simulink model "wlan_phy_rx_pmd"  (Simulink 7.8)
settings: Solver=VariableStepDiscrete  SolverMode=SingleTasking  StartTime=0.0  StopTime=rx_sim_time  RelTol=1e-3
PostLoadFcn:
  | wlan_phy_rx_init
InitFcn:
  | wlan_phy_rx_init
StartFcn:
  | wlan_phy_rx_init
values from neighbouring files (symbols this model uses):
  barker_seq20 = 0.95 * circshift(barker_seq20, [0 4]) ./ max(abs(barker_seq20))    [wlan_phy_rx_init.m]
  bit_scrambler_lfsr_bytes = bi2de(reshape(repmat(scr, 1, 8), 8, 127)', 'left-msb')    [wlan_phy_rx_init.m]
  deinterleave_ROM = [deinterleave_ROM interleave_64QAM zeros(1, 512-length(interleave_64QAM))]    [wlan_phy_rx_init.m]
  longCorr_coef_i = [3*real(longCorr_coef)]    [wlan_phy_rx_init.m]
  longCorr_coef_q = [3*imag(longCorr_coef)]    [wlan_phy_rx_init.m]
  MAX_CP_LEN = 32    [wlan_phy_rx_init.m]
  MAX_NCBPS = NCBPS_64QAM    (= 288)    [wlan_phy_rx_init.m]
  MAX_NUM_BYTES = 4096    [wlan_phy_rx_init.m]
  MAX_NUM_SC = 64    [wlan_phy_rx_init.m]
  MAX_NUM_SYMS = 600    [wlan_phy_rx_init.m]
  NCBPS_16QAM = 192    [wlan_phy_rx_init.m]
  NCBPS_64QAM = 288    [wlan_phy_rx_init.m]
  NCBPS_BPSK = 48    [wlan_phy_rx_init.m]
  NCBPS_QPSK = 96    [wlan_phy_rx_init.m]
  NDBPS_16QAM12 = 96    [wlan_phy_rx_init.m]
  NDBPS_16QAM34 = 144    [wlan_phy_rx_init.m]
  NDBPS_64QAM23 = 192    [wlan_phy_rx_init.m]
  NDBPS_64QAM34 = 216    [wlan_phy_rx_init.m]
  NDBPS_BPSK12 = 24    [wlan_phy_rx_init.m]
  NDBPS_BPSK34 = 36    [wlan_phy_rx_init.m]
  NDBPS_QPSK12 = 48    [wlan_phy_rx_init.m]
  NDBPS_QPSK34 = 72    [wlan_phy_rx_init.m]
  PHY_MIN_PKT_LEN = 14    [wlan_phy_rx_init.m]
  preFFT_sampBuff_numSamps = 4*MAX_NUM_SC    (= 256)    [wlan_phy_rx_init.m]
  rx_sim_sig_samp_time = 8    [wlan_phy_rx_init.m]
  scr_ind_rev = zeros(1,128)    [wlan_phy_rx_init.m]
undefined symbols (not defined in the file or in its folder): gcbh, xlBlockLoadCallback, xlGetNormalizedPeriod, xlipgetpartsetting
libraries: xbsIndex_r4 x3524, simulink x1, xbsEDKLib_r4 x1
(part 10 of 21)

== system wlan_phy_rx_pmd/Detect & Decode/Soft Demap/16QAM Soft De-Map ==
  note: 16-QAM Demap:
      | Each sym is [b0 b1 b2 b3]
      | b0 = 1 if (I > 0)
      | b1 = 1 if(abs(I) < 2/sqrt(10))
      | b2 = 1 if(Q > 0)
      | b3 = 1 if(abs(Q) < 2/sqrt(10))
  IQ Valid : Inport
  I : Inport  Port=2
  Q : Inport  Port=3
  Reest : Inport  Port=4
  Absolute : xbsIndex_r4/Absolute  precision=Full  arith_type=Unsigned  n_bits=8  bin_pt=0  quantization=Truncate  overflow=Saturate  en=off  latency=0  dbl_ovrd=off  block_type=abs
  AddSub : xbsIndex_r4/AddSub  mode=Subtraction  use_carryin=off  use_carryout=off  en=off  latency=0  precision=User Defined  arith_type="Signed  (2's comp)"  n_bits=8  bin_pt=7  quantization=Truncate  overflow=Saturate  dbl_ovrd=off  use_behavioral_HDL=off  hw_selection=Fabric  pipelined=off  block_type=addsub
  Constant : xbsIndex_r4/Constant  const=2/sqrt(10)  gui_display_data_type=Fixed-point  arith_type=Unsigned  n_bits=12  bin_pt=12  preci_type=Single  exp_width=8  frac_width=24  explicit_period=off  period=1  equ=P=C  opselect=C  inp2=PCIN>>17  opr=+  inp1=P  carry=CIN  dbl_ovrd=off  block_type=constant
  Delay2 : xbsIndex_r4/Delay  rst=off  infoeditControl=Selection of Reset will increase slice count due to use of real FFs and instead of SRLs  en=off  latency=1  dbl_ovrd=off  reg_retiming=off  block_type=delay
  Inverter : xbsIndex_r4/Inverter  en=off  latency=0  dbl_ovrd=off  block_type=inv
      settings: xl_area=[0 0 0 0 0 0 0]  block_version=VER_STRING_GOES_HERE
  Logical1 : xbsIndex_r4/Logical  logical_function=OR  inputs=2  en=off  latency=0  precision=Full  arith_type=Unsigned  n_bits=16  bin_pt=0  align_bp=on  dbl_ovrd=off  block_type=logical
  Logical2 : xbsIndex_r4/Logical  logical_function=OR  inputs=2  en=off  latency=0  precision=Full  arith_type=Unsigned  n_bits=16  bin_pt=0  align_bp=on  dbl_ovrd=off  block_type=logical
  Mux : xbsIndex_r4/Mux  inputs=2  en=off  latency=0  precision=Full  arith_type=Unsigned  n_bits=16  bin_pt=14  quantization=Truncate  overflow=Wrap  dbl_ovrd=off  block_type=mux
  Register1 : xbsIndex_r4/Register  init=0  rst=off  en=on  dbl_ovrd=off  block_type=register
  S-R Latch1 : Subsystem
  Ready : Outport
  Bit A : Outport  Port=2
  Bit B : Outport  Port=3
  Bits Valid : Outport  Port=4
  wires:
  IQ Valid/1 -> S-R Latch1/1(S), Register1/2, Logical2/1, Delay2/1
  S-R Latch1/1(Q) -> Inverter/1, Mux/1, Logical1/2
  Inverter/1 -> Ready/1
  Q/1 -> Register1/1
  Constant/1 -> AddSub/1
  Absolute/1 -> AddSub/2
  Mux/1 -> Bit A/1, Absolute/1
  I/1 -> Mux/2
  Register1/1 -> Mux/3
  AddSub/1 -> Bit B/1
  Delay2/1 -> Logical2/2
  Logical2/1 -> Bits Valid/1
  Logical1/1 -> S-R Latch1/2(R)
  Reest/1 -> Logical1/1

== system wlan_phy_rx_pmd/Detect & Decode/Soft Demap/64QAM Soft De-Map ==
  note: 64-QAM Demap:
      | Each sym is [b0 b1 b2 b3 b4 b5]
      | b0 = 1 if (I > 0)
      | b1 = 1 if(abs(I) < 4/sqrt(42))
      |
      | b2 = 1 if(2/sqrt(42) < abs(I) < 6/sqrt(42))
      | b3 = 1 if(Q > 0)
      |
      | b4 = 1 if(abs(Q) < 4/sqrt(42))
      | b5 = 1 if(2/sqrt(42) < abs(Q) < 6/sqrt(42))
  IQ Valid : Inport
  I : Inport  Port=2
  Q : Inport  Port=3
  Reest : Inport  Port=4
  64QAM Demap : Scope  NumInputPorts=8
      settings: TimeRange=20000  YMin=0~-1~0~0~-1~0~-1~-1  YMax=1~1~1~1~1~1~1~1  SaveName=ScopeData26
  Ctrl : Subsystem
  Gateway Out{1..6} (6 alike) : xbsIndex_r4/Gateway Out  inherit_from_input=off  hdl_port=off  timing_constraint=None  locs_specified=off  LOCs={}  UseAsDAC=off  DACChannel='1'  block_type=gatewayout
  Logical3 : xbsIndex_r4/Logical  logical_function=AND  inputs=2  en=off  latency=0  precision=Full  arith_type=Unsigned  n_bits=16  bin_pt=0  align_bp=on  dbl_ovrd=off  block_type=logical
  Mux : xbsIndex_r4/Mux  inputs=3  en=off  latency=0  precision=Full  arith_type=Unsigned  n_bits=16  bin_pt=14  quantization=Truncate  overflow=Wrap  dbl_ovrd=off  block_type=mux
  Mux1 : xbsIndex_r4/Mux  inputs=3  en=off  latency=0  precision=Full  arith_type=Unsigned  n_bits=16  bin_pt=14  quantization=Truncate  overflow=Wrap  dbl_ovrd=off  block_type=mux
  Register2 : xbsIndex_r4/Register  init=0  rst=off  en=on  dbl_ovrd=off  block_type=register
  Register3 : xbsIndex_r4/Register  init=0  rst=off  en=on  dbl_ovrd=off  block_type=register
  b1/b4 map : Subsystem
  b1/b4 map1 : Subsystem
  b2/b5 map : Subsystem
  b2/b5 map1 : Subsystem
  Ready : Outport
  Bit A : Outport  Port=2
  Bit B : Outport  Port=3
  Bits Valid : Outport  Port=4
  wires:
  I/1 -> Register2/1, Gateway Out3/1
  Mux/1 -> Bit A/1
  b1/b4 map/1(b) -> Mux1/2
  Logical3/1 -> Register2/2, Register3/2
  IQ Valid/1 -> Logical3/2, Ctrl/1(Vin), Gateway Out1/1
  b2/b5 map/1(b) -> Mux/3
  Register2/1 -> b2/b5 map/1(IQ), Mux/2, b1/b4 map/1(IQ)
  Register3/1 -> Mux1/3, b1/b4 map1/1(IQ), b2/b5 map1/1(IQ)
  Q/1 -> Register3/1, Gateway Out2/1
  b1/b4 map1/1(b) -> Mux/4
  b2/b5 map1/1(b) -> Mux1/4
  Mux1/1 -> Bit B/1
  Ctrl/3(Sel) -> Mux/1, Mux1/1, Gateway Out4/1
  Ctrl/2(Vout) -> Bits Valid/1, Gateway Out5/1
  Ctrl/1(Ready) -> Logical3/1, Ready/1, Gateway Out6/1
  Gateway Out1/1 -> 64QAM Demap/1  "valid in"
  Gateway Out3/1 -> 64QAM Demap/2  "I In"
  Gateway Out2/1 -> 64QAM Demap/3  "Q In"
  Gateway Out4/1 -> 64QAM Demap/4  "Mux Sel"
  Gateway Out5/1 -> 64QAM Demap/5  "Vout"
  Gateway Out6/1 -> 64QAM Demap/6  "Ready"
  Reest/1 -> Ctrl/2(Reest)

== system wlan_phy_rx_pmd/Detect & Decode/Soft Demap/BPSK Soft De-Map ==
  V_in : Inport
  I : Inport  Port=2
  Q : Inport  Port=3
  Reest : Inport  Port=4
  Constant : xbsIndex_r4/Constant  const=1  gui_display_data_type=Boolean  arith_type=Boolean  n_bits=16  bin_pt=14  preci_type=Single  exp_width=8  frac_width=24  explicit_period=on  period=1  equ=P=C  opselect=C  inp2=PCIN>>17  opr=+  inp1=P  carry=CIN  dbl_ovrd=off  block_type=constant
  Logical1 : xbsIndex_r4/Logical  logical_function=OR  inputs=2  en=off  latency=0  precision=Full  arith_type=Unsigned  n_bits=16  bin_pt=0  align_bp=on  dbl_ovrd=off  block_type=logical
  Logical2 : xbsIndex_r4/Logical  logical_function=AND  inputs=2  en=off  latency=0  precision=Full  arith_type=Unsigned  n_bits=16  bin_pt=0  align_bp=on  dbl_ovrd=off  block_type=logical
  Register2 : xbsIndex_r4/Register  init=0  rst=off  en=on  dbl_ovrd=off  block_type=register
  S-R Latch1 : Subsystem
  Ready : Outport
  Bit A : Outport  Port=2
  Bit B : Outport  Port=3
  Bits Valid : Outport  Port=4
  wires:
  I/1 -> Register2/1, Bit B/1
  Constant/1 -> Ready/1
  V_in/1 -> S-R Latch1/1(S), Register2/2, Logical2/2
  S-R Latch1/1(Q) -> Logical2/1
  Register2/1 -> Bit A/1
  Logical2/1 -> Bits Valid/1, Logical1/2
  Logical1/1 -> S-R Latch1/2(R)
  Reest/1 -> Logical1/1

== system wlan_phy_rx_pmd/Detect & Decode/Soft Demap/QPSK Soft De-Map ==
  IQ Valid : Inport
  I : Inport  Port=2
  Q : Inport  Port=3
  Reest : Inport  Port=4
  Constant1 : xbsIndex_r4/Constant  const=1  gui_display_data_type=Fixed-point  arith_type=Boolean  n_bits=1  bin_pt=0  preci_type=Single  exp_width=8  frac_width=24  explicit_period=off  period=1  equ=P=C  opselect=C  inp2=PCIN>>17  opr=+  inp1=P  carry=CIN  dbl_ovrd=off  block_type=constant
      settings: block_version=VER_STRING_GOES_HERE
  Ready : Outport
  Bit A : Outport  Port=2
  Bit B : Outport  Port=3
  Bits Valid : Outport  Port=4
  wires:
  I/1 -> Bit A/1
  Q/1 -> Bit B/1
  IQ Valid/1 -> Bits Valid/1
  Constant1/1 -> Ready/1
  Reest/1 -> ?

== system wlan_phy_rx_pmd/Detect & Decode/Soft Demap/Scale & Quantize ==
  Bit A : Inport
  Bit B : Inport  Port=2
  Bits Valid : Inport  Port=3
  mod_sel : Inport  Port=4
  Delay : xbsIndex_r4/Delay  rst=off  infoeditControl=Selection of Reset will increase slice count due to use of real FFs and instead of SRLs  en=off  latency=1  dbl_ovrd=off  reg_retiming=off  block_type=delay
  Delay1 : xbsIndex_r4/Delay  rst=off  infoeditControl=Selection of Reset will increase slice count due to use of real FFs and instead of SRLs  en=off  latency=1  dbl_ovrd=off  reg_retiming=off  block_type=delay
  Display : Display
  Display1 : Display
  Display2 : Display
  Gateway Out{1..8} (8 alike) : xbsIndex_r4/Gateway Out  inherit_from_input=off  hdl_port=off  timing_constraint=None  locs_specified=off  LOCs={}  UseAsDAC=off  DACChannel='1'  block_type=gatewayout
  Mult1 : xbsIndex_r4/Mult  precision=User Defined  arith_type="Signed  (2's comp)"  n_bits=13  bin_pt=0  quantization=Truncate  overflow=Saturate  en=off  latency=1  dbl_ovrd=off  use_behavioral_HDL=off  opt=Speed  use_embedded=on  optimum_pipeline=off  pipeline=on  use_rpm=on  placement_style=Triangular  block_type=mult
  Mult2 : xbsIndex_r4/Mult  precision=User Defined  arith_type="Signed  (2's comp)"  n_bits=13  bin_pt=0  quantization=Truncate  overflow=Saturate  en=off  latency=1  dbl_ovrd=off  use_behavioral_HDL=off  opt=Speed  use_embedded=on  optimum_pipeline=off  pipeline=on  use_rpm=on  placement_style=Triangular  block_type=mult
  Mux : xbsIndex_r4/Mux  inputs=4  en=off  latency=0  precision=Full  arith_type=Unsigned  n_bits=16  bin_pt=14  quantization=Truncate  overflow=Wrap  dbl_ovrd=off  block_type=mux
  Negate : xbsIndex_r4/Negate  precision=User Defined  arith_type="Signed  (2's comp)"  n_bits=4  bin_pt=0  quantization=Truncate  overflow=Saturate  en=off  latency=0  dbl_ovrd=off  block_type=negate
  Negate1 : xbsIndex_r4/Negate  precision=User Defined  arith_type="Signed  (2's comp)"  n_bits=4  bin_pt=0  quantization=Truncate  overflow=Saturate  en=off  latency=0  dbl_ovrd=off  block_type=negate
  Quantize : Subsystem
  Quantize1 : Subsystem
  Reinterpret : xbsIndex_r4/Reinterpret  force_arith_type=off  arith_type="Signed  (2's comp)"  force_bin_pt=on  bin_pt=0  block_type=reinterpret
  Reinterpret1 : xbsIndex_r4/Reinterpret  force_arith_type=off  arith_type="Signed  (2's comp)"  force_bin_pt=on  bin_pt=0  block_type=reinterpret
  Scale/Quant : Scope  NumInputPorts=8
      settings: TimeRange=178.0593691607082  YMin=-1~-95.55~5.7~-573.3~-4.2~-1~-1~-1  YMax=1~-86.45~6.3~-518.7~-3.8~1~1~1  SaveName=ScopeData2
  LLR A : Outport
  LLR B : Outport  Port=2
  LLRs Valid : Outport  Port=3
  " mod_sel" : Outport  Port=4
  wires:
  mod_sel/1 -> Mux/1, Delay1/1
  [regRx_FEC_SCALE_QPSK] -> Mux/3, Mux/2, Gateway Out6/1
  [regRx_FEC_SCALE_16QAM] -> Mux/4, Gateway Out7/1
  [regRx_FEC_SCALE_64QAM] -> Mux/5, Gateway Out8/1
  Bit A/1 -> Reinterpret/1
  Mult1/1 -> Quantize/1(D), Gateway Out1/1
  Quantize/1(Q) -> Gateway Out5/1, Negate/1
  Mux/1 -> Mult1/2, Gateway Out4/1, Mult2/2
  Bits Valid/1 -> Delay/1, Gateway Out2/1
  Delay/1 -> LLRs Valid/1
  Delay1/1 -> " mod_sel"/1
  Gateway Out2/1 -> Scale/Quant/1  "In Valid"
  Gateway Out3/1 -> Scale/Quant/2  "Soft Bit In"
  Gateway Out4/1 -> Scale/Quant/3  "Scale"
  Gateway Out1/1 -> Scale/Quant/4  "Bit Scaled"
  Gateway Out5/1 -> Scale/Quant/5  "LLR"
  Reinterpret/1 -> Mult1/1, Gateway Out3/1
  Negate/1 -> LLR A/1
  Gateway Out8/1 -> Display2/1
  Gateway Out7/1 -> Display1/1
  Gateway Out6/1 -> Display/1
  Bit B/1 -> Reinterpret1/1
  Reinterpret1/1 -> Mult2/1
  Mult2/1 -> Quantize1/1(D)
  Quantize1/1(Q) -> Negate1/1
  Negate1/1 -> LLR B/1

== system wlan_phy_rx_pmd/Detect & Decode/Sym FIFO/S-R Latch1 ==
  S : Inport
  R : Inport  Port=2
  Constant1 : xbsIndex_r4/Constant  const=1  gui_display_data_type=Fixed-point  arith_type=Boolean  n_bits=1  bin_pt=0  preci_type=Single  exp_width=8  frac_width=24  explicit_period=off  period=1  equ=P=C  opselect=C  inp2=PCIN>>17  opr=+  inp1=P  carry=CIN  dbl_ovrd=off  block_type=constant
      settings: block_version=VER_STRING_GOES_HERE
  Convert1 : xbsIndex_r4/Convert  gui_display_data_type=Boolean  arith_type=Boolean  n_bits=1  bin_pt=0  float_type=Single  exp_bits=8  fraction_bits=24  quantization=Truncate  overflow=Wrap  en=off  latency=0  dbl_ovrd=off  pipeline=off  block_type=convert
  Convert2 : xbsIndex_r4/Convert  gui_display_data_type=Boolean  arith_type=Boolean  n_bits=1  bin_pt=0  float_type=Single  exp_bits=8  fraction_bits=24  quantization=Truncate  overflow=Wrap  en=off  latency=0  dbl_ovrd=off  pipeline=off  block_type=convert
  Register2 : xbsIndex_r4/Register  init=0  rst=on  en=on  dbl_ovrd=off  block_type=register
      settings: xl_area=[1 1 0 0 0 0 0]  block_version=VER_STRING_GOES_HERE
  Q : Outport
  wires:
  S/1 -> Convert2/1
  Register2/1 -> Q/1
  R/1 -> Convert1/1
  Constant1/1 -> Register2/1
  Convert1/1 -> Register2/2
  Convert2/1 -> Register2/3

== system wlan_phy_rx_pmd/FCS & Pkt Buf/FCS Calc/CRC32 Calc ==
  byte_valid : Inport
  byte : Inport  Port=2
  rst : Inport  Port=3
  24LSB : xbsIndex_r4/Slice  nbits=24  boolean_output=off  mode=Lower Bit Location + Width  bit1=0  base1=MSB of Input  bit0=0  base0=LSB of Input  dbl_ovrd=off  block_type=slice
      settings: infoedit="Extracts a given range of bits from each input sample and presents it at the output.  The output type is ordinarily unsigned with binary point at zero, but can be Boolean when the slice is one bit wide.<P><P>Hardware notes: In hardware this block costs nothing."  block_version=VER_STRING_GOES_HERE
  8MSB : xbsIndex_r4/Slice  nbits=8  boolean_output=off  mode=Upper Bit Location + Width  bit1=0  base1=MSB of Input  bit0=0  base0=LSB of Input  dbl_ovrd=off  block_type=slice
      settings: infoedit="Extracts a given range of bits from each input sample and presents it at the output.  The output type is ordinarily unsigned with binary point at zero, but can be Boolean when the slice is one bit wide.<P><P>Hardware notes: In hardware this block costs nothing."  block_version=VER_STRING_GOES_HERE
  Assert1 : xbsIndex_r4/Assert  assert_type=off  type_source=Explicitly  gui_display_data_type=Fixed-point  arith_type="Signed  (2's comp)"  n_bits=16  bin_pt=14  preci_type=Single  exp_width=8  frac_width=24  assert_rate=on  rate_source=Explicitly  period=1  output_port=on  block_type=assert
      settings: block_version=VER_STRING_GOES_HERE
  CRC Remainders1 : xbsIndex_r4/ROM  depth=256  initVector=CRC_Table32  distributed_mem=Distributed memory  rst=off  init_reg=0  en=off  latency=0  gui_display_data_type=Fixed-point  arith_type=Unsigned  n_bits=32  bin_pt=0  preci_type=Single  exp_width=8  frac_width=24  dbl_ovrd=off  optimize=Area  use_rpm=off  block_type=sprom
      settings: xl_area=[0, 0, 0, 0, 0, 0, 0]  block_version=VER_STRING_GOES_HERE
  Concat1 : xbsIndex_r4/Concat  num_inputs=2  dbl_ovrd=off  block_type=concat
      settings: block_version=VER_STRING_GOES_HERE
  Constant1 : xbsIndex_r4/Constant  const=0  gui_display_data_type=Fixed-point  arith_type=Unsigned  n_bits=8  bin_pt=0  preci_type=Single  exp_width=8  frac_width=24  explicit_period=off  period=1  equ=P=C  opselect=C  inp2=PCIN>>17  opr=+  inp1=P  carry=CIN  dbl_ovrd=off  block_type=constant
      settings: block_version=VER_STRING_GOES_HERE
  Constant3 : xbsIndex_r4/Constant  const=hex2dec('C704DD7B')  gui_display_data_type=Fixed-point  arith_type=Unsigned  n_bits=32  bin_pt=0  preci_type=Single  exp_width=8  frac_width=24  explicit_period=off  period=1  equ=P=C  opselect=C  inp2=PCIN>>17  opr=+  inp1=P  carry=CIN  dbl_ovrd=off  block_type=constant
  Endian Swap : Subsystem
  FCS Calc : Scope  NumInputPorts=8
      settings: TimeRange=10000  YMin=0~0~10~-1~-1~-1~-1~-1  YMax=1~1~120~1~1~1~1~1  SaveName=ScopeData15
  Gateway Out{1..3,10} (4 alike) : xbsIndex_r4/Gateway Out  inherit_from_input=off  hdl_port=off  timing_constraint=None  locs_specified=off  LOCs={}  UseAsDAC=off  DACChannel='1'  block_type=gatewayout
  Logical3 : xbsIndex_r4/Logical  logical_function=XOR  inputs=2  en=off  latency=0  precision=Full  arith_type=Unsigned  n_bits=8  bin_pt=2  align_bp=on  dbl_ovrd=off  block_type=logical
      settings: xl_area=[0, 0, 0, 0, 0, 0, 0]  block_version=VER_STRING_GOES_HERE
  Logical4 : xbsIndex_r4/Logical  logical_function=XOR  inputs=2  en=off  latency=0  precision=Full  arith_type=Unsigned  n_bits=8  bin_pt=2  align_bp=on  dbl_ovrd=off  block_type=logical
      settings: xl_area=[0, 0, 0, 0, 0, 0, 0]  block_version=VER_STRING_GOES_HERE
  Relational1 : xbsIndex_r4/Relational  mode=a=b  en=off  latency=0  dbl_ovrd=off  block_type=relational
  crc_accum : xbsIndex_r4/Register  init=hex2dec('FFFFFFFF')  rst=on  en=on  dbl_ovrd=off  block_type=register
      settings: xl_area=[0, 0, 0, 0, 0, 0, 0]  block_version=VER_STRING_GOES_HERE
  FCS Good : Outport
  wires:
  Endian Swap/1(I) -> Logical3/2
  Logical3/1 -> CRC Remainders1/1
  CRC Remainders1/1 -> Logical4/2
  Concat1/1 -> Logical4/1
  Constant1/1 -> Concat1/2
  Logical4/1 -> crc_accum/1
  crc_accum/1 -> Assert1/1
  Assert1/1 -> 24LSB/1, 8MSB/1, Relational1/1
  byte_valid/1 -> crc_accum/3, Gateway Out10/1
  rst/1 -> crc_accum/2, Gateway Out1/1
  24LSB/1 -> Concat1/1
  8MSB/1 -> Logical3/1
  byte/1 -> Endian Swap/1(B), Gateway Out2/1
  Constant3/1 -> Relational1/2
  Relational1/1 -> FCS Good/1, Gateway Out3/1
  Gateway Out10/1 -> FCS Calc/1  "Vin"
  Gateway Out1/1 -> FCS Calc/2  "Reset"
  Gateway Out2/1 -> FCS Calc/3  "Byte"
  Gateway Out3/1 -> FCS Calc/4  "Good"

== system wlan_phy_rx_pmd/FCS & Pkt Buf/FCS Calc/Posedge ==
  D : Inport
  Delay : xbsIndex_r4/Delay  rst=off  infoeditControl=Selection of Reset will increase slice count due to use of real FFs and instead of SRLs  en=off  latency=1  dbl_ovrd=off  reg_retiming=off  block_type=delay
      settings: infoedit=Hardware notes: A delay line is a chain, each link of which is an SRL16 followed by a flip-flop. If register retiming is enabled, the delay line is a chain of flip-flops.  xl_area=[1 1 0 0 0 0 0]  block_version=VER_STRING_GOES_HERE
  Inverter : xbsIndex_r4/Inverter  en=off  latency=0  dbl_ovrd=off  block_type=inv
      settings: xl_area=[0 0 0 0 0 0 0]  block_version=VER_STRING_GOES_HERE
  Logical1 : xbsIndex_r4/Logical  logical_function=AND  inputs=2  en=off  latency=0  precision=Full  arith_type=Unsigned  n_bits=8  bin_pt=2  align_bp=on  dbl_ovrd=off  block_type=logical
      settings: xl_area=[1 0 0 1 0 0 0]  block_version=VER_STRING_GOES_HERE
  Q : Outport
  wires:
  D/1 -> Logical1/2, Delay/1
  Logical1/1 -> Q/1
  Inverter/1 -> Logical1/1
  Delay/1 -> Inverter/1

== system wlan_phy_rx_pmd/FCS & Pkt Buf/Pkt Buf Interface/Addr Check ==
  note: The pkt buf interface logic concatenates the PHY-generated byte address
      | with the pkt buf index and MAC-specified meta-data offset. If the PHY
      | attempts to write a byte index larger than the actual pkt buf space the
      | address will wrap, overwriting the meta-data at the head of the pkt buf.
      | This subsystem prevents this by disabling the Write En for byte addresses
      | larger than a software-specified value. The MAC should set this value
      | based on its knowledge of the pkt buf size and meta-data size.
  WrEn : Inport
  Addr : Inport  Port=2
  Logical1 : xbsIndex_r4/Logical  logical_function=AND  inputs=2  en=off  latency=0  precision=Full  arith_type=Unsigned  n_bits=8  bin_pt=2  align_bp=on  dbl_ovrd=off  block_type=logical
      settings: xl_area=[0, 0, 0, 0, 0, 0, 0]  block_version=VER_STRING_GOES_HERE
  Relational : xbsIndex_r4/Relational  mode=a<b  en=off  latency=0  dbl_ovrd=off  block_type=relational
  " WrEn" : Outport
  " Addr" : Outport  Port=2
  wires:
  [regRx_PktBuf_Max_WriteAddr] -> Relational/2
  Relational/1 -> Logical1/2
  WrEn/1 -> Logical1/1
  Logical1/1 -> " WrEn"/1
  Addr/1 -> Relational/1, " Addr"/1

== system wlan_phy_rx_pmd/FCS & Pkt Buf/Pkt Buf Interface/BRAM IF 64b ==
  note: Word Addr
      | (1K words max)
  "byte " : Inport
  byte_valid : Inport  Port=2
  byte_ind : Inport  Port=3
  Pkt Buf Sel : Inport  Port=4
  9LSB + 3 : xbsIndex_r4/Slice  nbits=9  boolean_output=off  mode=Lower Bit Location + Width  bit1=2  base1=MSB of Input  bit0=3  base0=LSB of Input  dbl_ovrd=off  block_type=slice
      settings: block_version=8.2.02
  AddSub1 : xbsIndex_r4/AddSub  mode=Addition  use_carryin=off  use_carryout=off  en=off  latency=0  precision=User Defined  arith_type=Unsigned  n_bits=15  bin_pt=0  quantization=Truncate  overflow=Wrap  dbl_ovrd=off  use_behavioral_HDL=off  hw_selection=Fabric  pipelined=off  block_type=addsub
  AddSub2 : xbsIndex_r4/AddSub  mode=Addition  use_carryin=off  use_carryout=off  en=off  latency=0  precision=User Defined  arith_type=Unsigned  n_bits=15  bin_pt=0  quantization=Truncate  overflow=Wrap  dbl_ovrd=off  use_behavioral_HDL=off  hw_selection=Fabric  pipelined=off  block_type=addsub
  BRAM I/O : Subsystem
  BRAM WEn Decoding : Subsystem
  Concat : xbsIndex_r4/Concat  num_inputs=4  dbl_ovrd=off  block_type=concat
      settings: block_version=8.2.02
  Concat1 : xbsIndex_r4/Concat  num_inputs=4  dbl_ovrd=off  block_type=concat
      settings: block_version=8.2.02
  Concat{2..4,6..7} (5 alike) : xbsIndex_r4/Concat  num_inputs=2  dbl_ovrd=off  block_type=concat
      settings: block_version=8.2.02
  Concat5 : xbsIndex_r4/Concat  num_inputs=4  dbl_ovrd=off  block_type=concat
      settings: block_version=8.2.02
  Constant1 : xbsIndex_r4/Constant  const=0  gui_display_data_type=Fixed-point  arith_type=Unsigned  n_bits=2  bin_pt=0  preci_type=Single  exp_width=8  frac_width=24  explicit_period=off  period=1  equ=P=C  opselect=C  inp2=PCIN>>17  opr=+  inp1=P  carry=CIN  dbl_ovrd=off  block_type=constant
  Constant2 : xbsIndex_r4/Constant  const=0  gui_display_data_type=Fixed-point  arith_type=Unsigned  n_bits=4  bin_pt=0  preci_type=Single  exp_width=8  frac_width=24  explicit_period=off  period=1  equ=P=C  opselect=C  inp2=PCIN>>17  opr=+  inp1=P  carry=CIN  dbl_ovrd=off  block_type=constant
  Constant3 : xbsIndex_r4/Constant  const=0  gui_display_data_type=Fixed-point  arith_type=Unsigned  n_bits=16  bin_pt=0  preci_type=Single  exp_width=8  frac_width=24  explicit_period=on  period=1  equ=P=C  opselect=C  inp2=PCIN>>17  opr=+  inp1=P  carry=CIN  dbl_ovrd=off  block_type=constant
      settings: block_version=8.2.02
  Constant4 : xbsIndex_r4/Constant  const=0  gui_display_data_type=Fixed-point  arith_type=Unsigned  n_bits=3  bin_pt=0  preci_type=Single  exp_width=8  frac_width=24  explicit_period=off  period=1  equ=P=C  opselect=C  inp2=PCIN>>17  opr=+  inp1=P  carry=CIN  dbl_ovrd=off  block_type=constant
  Constant5 : xbsIndex_r4/Constant  const=0  gui_display_data_type=Fixed-point  arith_type=Unsigned  n_bits=3  bin_pt=0  preci_type=Single  exp_width=8  frac_width=24  explicit_period=on  period=1  equ=P=C  opselect=C  inp2=PCIN>>17  opr=+  inp1=P  carry=CIN  dbl_ovrd=off  block_type=constant
      settings: block_version=8.2.02
  Constant7 : xbsIndex_r4/Constant  const=0  gui_display_data_type=Fixed-point  arith_type=Unsigned  n_bits=3  bin_pt=0  preci_type=Single  exp_width=8  frac_width=24  explicit_period=off  period=1  equ=P=C  opselect=C  inp2=PCIN>>17  opr=+  inp1=P  carry=CIN  dbl_ovrd=off  block_type=constant
  Convert : xbsIndex_r4/Convert  gui_display_data_type=Fixed-point  arith_type=Unsigned  n_bits=8  bin_pt=0  float_type=Single  exp_bits=8  fraction_bits=24  quantization=Truncate  overflow=Wrap  en=off  latency=0  dbl_ovrd=off  pipeline=off  block_type=convert
  Display : Display
  Gateway Out{1..8,10} (9 alike) : xbsIndex_r4/Gateway Out  inherit_from_input=off  hdl_port=off  timing_constraint=None  locs_specified=off  LOCs={}  UseAsDAC=off  DACChannel='1'  block_type=gatewayout
  LSB : xbsIndex_r4/Slice  nbits=1  boolean_output=off  mode=Lower Bit Location + Width  bit1=0  base1=MSB of Input  bit0=0  base0=LSB of Input  dbl_ovrd=off  block_type=slice
      settings: block_version=8.2.02
  Logical : xbsIndex_r4/Logical  logical_function=AND  inputs=2  en=off  latency=0  precision=Full  arith_type=Unsigned  n_bits=16  bin_pt=0  align_bp=on  dbl_ovrd=off  block_type=logical
      settings: block_version=8.2.02
  Logical4 : xbsIndex_r4/Logical  logical_function=XOR  inputs=2  en=off  latency=0  precision=Full  arith_type=Unsigned  n_bits=16  bin_pt=0  align_bp=on  dbl_ovrd=off  block_type=logical
  Mux{,1..3} (4 alike) : xbsIndex_r4/Mux  inputs=2  en=off  latency=0  precision=Full  arith_type=Unsigned  n_bits=16  bin_pt=14  quantization=Truncate  overflow=Wrap  dbl_ovrd=off  block_type=mux
  Pkt Buf Addr : Scope  NumInputPorts=8
      settings: TimeRange=10000  YMin=0~0~0~0~0~-1~-1~-1  YMax=17.5~150~17.5~1~4.5e+009~1~1~1  SaveName=ScopeData27
  wires:
  Gateway Out4/1 -> Pkt Buf Addr/4  "Rx Byte Valid"
  9LSB + 3/1 -> Concat1/3
  byte_valid/1 -> BRAM WEn Decoding/2(byte_valid), Gateway Out4/1
  AddSub2/1 -> Mux1/2, BRAM WEn Decoding/1(byte_addr), Gateway Out2/1
  Pkt Buf Sel/1 -> Concat1/2
  "byte "/1 -> Concat/1, Concat/2, Concat/3, Concat/4
  Gateway Out2/1 -> Pkt Buf Addr/3  "Rx Byte Index"
  Gateway Out1/1 -> Pkt Buf Addr/2  "BRAM WEN"
  Gateway Out10/1 -> Pkt Buf Addr/1  "BRAM Addr"
  Constant3/1 -> Concat1/1
  Constant5/1 -> Concat1/4
  Concat1/1 -> BRAM I/O/2(Addr[31:0]), Gateway Out10/1
  BRAM WEn Decoding/1(WrEn[7:0]) -> Mux/2
  byte_ind/1 -> AddSub2/1
  Concat2/1 -> AddSub2/2
  [regRx_PktBuf_Rx_Addr_Offset] -> Concat2/1
  Constant7/1 -> Concat2/2
  Gateway Out3/1 -> Pkt Buf Addr/8  "H Est Valid"
  Mux2/1 -> Concat3/1, Concat3/2, Gateway Out7/1
  Concat/1 -> Mux2/2
  [CHAN_EST_IND] -> Concat4/1, LSB/1, Gateway Out5/1
  Constant1/1 -> Concat4/2
  Mux1/1 -> 9LSB + 3/1
  Logical/1 -> Mux2/1, Mux1/1, Mux/1
  Mux3/1 -> Mux/3, Gateway Out6/1
  [CHAN_EST_VALID] -> Concat5/1, Concat5/2, Concat5/3, Concat5/4, Gateway Out3/1
  Concat5/1 -> Concat6/1, Convert/1
  Constant2/1 -> Concat6/2
  [regRx_Record_Chan_Est_Pkt_Buf] -> Logical/1
  [CHAN_EST_VALID] -> Logical/2
  Concat4/1 -> AddSub1/2
  Concat7/1 -> AddSub1/1
  [regRx_PktBuf_Rx_H_Est_Offset] -> Concat7/1, Gateway Out8/1
  Constant4/1 -> Concat7/2
  AddSub1/1 -> Mux1/3
  Concat3/1 -> BRAM I/O/1(Dout[63:0])
  Mux/1 -> BRAM I/O/3(WEn[7:0]), Gateway Out1/1
  Concat6/1 -> Mux3/3
  Convert/1 -> Mux3/2
  [regRx_PKT_BUF_H_EST_ORDER_SWAP] -> Logical4/1
  LSB/1 -> Logical4/2
  Logical4/1 -> Mux3/1
  Gateway Out5/1 -> Pkt Buf Addr/7  "H Est Ind"
  Gateway Out6/1 -> Pkt Buf Addr/6  "H Est WrEn"
  [CHAN_EST_IQ_32b] -> Mux2/3
  Gateway Out7/1 -> Pkt Buf Addr/5  "Dout 32"
  Gateway Out8/1 -> Display/1

== system wlan_phy_rx_pmd/FCS & Pkt Buf/Pkt Buf Interface/DSSS Addr Calc ==
  note: DSSS Byte Index Rx -> Memory mapping
      |
      | R=Rx byte index
      | M=Memory byte index
      |
      | R  M
      | 0  0   SIGNAL[0]
      | 1  1   SERVICE[0]
      | 2  2   LENGTH[0]
      | 3  3   LENGTH[1]
      | 4  4   CRC16[0]
      | 5  5   CRC16[1]
      |
      | 6  8   MPDU[0]
      | 7  9   MPDU[1]
      | 8  10  MPDU[2]
      | ...
  R : Inport
  AddSub1 : xbsIndex_r4/AddSub  mode=Addition  use_carryin=off  use_carryout=off  en=off  latency=0  precision=Full  arith_type=Unsigned  n_bits=3  bin_pt=0  quantization=Truncate  overflow=Wrap  dbl_ovrd=off  use_behavioral_HDL=off  hw_selection=Fabric  pipelined=off  block_type=addsub
  Constant1 : xbsIndex_r4/Constant  const=5  gui_display_data_type=Fixed-point  arith_type=Unsigned  n_bits=3  bin_pt=0  preci_type=Single  exp_width=8  frac_width=24  explicit_period=off  period=1  equ=P=C  opselect=C  inp2=PCIN>>17  opr=+  inp1=P  carry=CIN  dbl_ovrd=off  block_type=constant
  Constant3 : xbsIndex_r4/Constant  const=2  gui_display_data_type=Fixed-point  arith_type=Unsigned  n_bits=3  bin_pt=0  preci_type=Single  exp_width=8  frac_width=24  explicit_period=off  period=1  equ=P=C  opselect=C  inp2=PCIN>>17  opr=+  inp1=P  carry=CIN  dbl_ovrd=off  block_type=constant
  Mux5 : xbsIndex_r4/Mux  inputs=2  en=off  latency=0  precision=Full  arith_type=Unsigned  n_bits=16  bin_pt=14  quantization=Truncate  overflow=Wrap  dbl_ovrd=off  block_type=mux
  Relational : xbsIndex_r4/Relational  mode=a<=b  en=off  latency=0  dbl_ovrd=off  block_type=relational
  M : Outport
  wires:
  Mux5/1 -> M/1
  Constant1/1 -> Relational/2
  Relational/1 -> Mux5/1
  R/1 -> Relational/1, AddSub1/1, Mux5/3
  Constant3/1 -> AddSub1/2
  AddSub1/1 -> Mux5/2

== system wlan_phy_rx_pmd/FCS & Pkt Buf/Pkt Buf Interface/MPDU Byte Outputs ==
  note: Byte index output to MAC is
      | indexed so 0 = MPDU start
      | (0x8 offset into pkt buffer)
  note: Outputs to MAC hw
  "Byte " : Inport
  Byte Valid : Inport  Port=2
  Byte Ind : Inport  Port=3
  AddSub1 : xbsIndex_r4/AddSub  mode=Subtraction  use_carryin=off  use_carryout=off  en=off  latency=0  precision=User Defined  arith_type=Unsigned  n_bits=14  bin_pt=0  quantization=Truncate  overflow=Saturate  dbl_ovrd=off  use_behavioral_HDL=off  hw_selection=Fabric  pipelined=off  block_type=addsub
  Constant4 : xbsIndex_r4/Constant  const=8  gui_display_data_type=Fixed-point  arith_type=Unsigned  n_bits=4  bin_pt=0  preci_type=Single  exp_width=8  frac_width=24  explicit_period=off  period=1  equ=P=C  opselect=C  inp2=PCIN>>17  opr=+  inp1=P  carry=CIN  dbl_ovrd=off  block_type=constant
  Logical4 : xbsIndex_r4/Logical  logical_function=AND  inputs=2  en=off  latency=0  precision=Full  arith_type=Unsigned  n_bits=16  bin_pt=0  align_bp=on  dbl_ovrd=off  block_type=logical
  Relational2 : xbsIndex_r4/Relational  mode=a>=b  en=off  latency=0  dbl_ovrd=off  block_type=relational
  tag scope: CUR_MPDU_BYTE=global, CUR_MPDU_BYTE_INDEX=global, CUR_MPDU_BYTE_VALID=global
  wires:
  Logical4/1 -> [CUR_MPDU_BYTE_VALID]
  Relational2/1 -> Logical4/1
  AddSub1/1 -> [CUR_MPDU_BYTE_INDEX]
  Constant4/1 -> AddSub1/2, Relational2/2
  Byte Ind/1 -> AddSub1/1, Relational2/1
  Byte Valid/1 -> Logical4/2
  "Byte "/1 -> [CUR_MPDU_BYTE]

== system wlan_phy_rx_pmd/FCS & Pkt Buf/Pkt Buf Interface/OFDM Addr Calc ==
  note: OFDM Byte Index Rx -> Memory mapping
      |
      | R=Rx byte index
      | M=Memory byte index
      |
      | R  M
      | 0  0   SIGNAL[0]
      | 1  1   SIGNAL[1]
      | 2  2   SIGNAL[2]
      | 3  3   SERVICE[0]
      | 4  4   SERVICE[1]
      |
      | 5  8   MPDU[0]
      | 6  9   MPDU[1]
      | 7  10  MPDU[2]
      | ...
  R : Inport
  AddSub1 : xbsIndex_r4/AddSub  mode=Addition  use_carryin=off  use_carryout=off  en=off  latency=0  precision=Full  arith_type=Unsigned  n_bits=3  bin_pt=0  quantization=Truncate  overflow=Wrap  dbl_ovrd=off  use_behavioral_HDL=off  hw_selection=Fabric  pipelined=off  block_type=addsub
  Constant1 : xbsIndex_r4/Constant  const=4  gui_display_data_type=Fixed-point  arith_type=Unsigned  n_bits=3  bin_pt=0  preci_type=Single  exp_width=8  frac_width=24  explicit_period=off  period=1  equ=P=C  opselect=C  inp2=PCIN>>17  opr=+  inp1=P  carry=CIN  dbl_ovrd=off  block_type=constant
  Constant3 : xbsIndex_r4/Constant  const=3  gui_display_data_type=Fixed-point  arith_type=Unsigned  n_bits=3  bin_pt=0  preci_type=Single  exp_width=8  frac_width=24  explicit_period=off  period=1  equ=P=C  opselect=C  inp2=PCIN>>17  opr=+  inp1=P  carry=CIN  dbl_ovrd=off  block_type=constant
  Mux5 : xbsIndex_r4/Mux  inputs=2  en=off  latency=0  precision=Full  arith_type=Unsigned  n_bits=16  bin_pt=14  quantization=Truncate  overflow=Wrap  dbl_ovrd=off  block_type=mux
  Relational : xbsIndex_r4/Relational  mode=a<=b  en=off  latency=0  dbl_ovrd=off  block_type=relational
  M : Outport
  wires:
  Constant3/1 -> AddSub1/2
  R/1 -> Relational/1, AddSub1/1, Mux5/3
  Relational/1 -> Mux5/1
  Constant1/1 -> Relational/2
  Mux5/1 -> M/1
  AddSub1/1 -> Mux5/2

== system wlan_phy_rx_pmd/FFT/Reset Gen/S-R Latch1 ==
  S : Inport
  R : Inport  Port=2
  Constant1 : xbsIndex_r4/Constant  const=1  gui_display_data_type=Fixed-point  arith_type=Boolean  n_bits=1  bin_pt=0  preci_type=Single  exp_width=8  frac_width=24  explicit_period=off  period=1  equ=P=C  opselect=C  inp2=PCIN>>17  opr=+  inp1=P  carry=CIN  dbl_ovrd=off  block_type=constant
      settings: block_version=VER_STRING_GOES_HERE
  Convert1 : xbsIndex_r4/Convert  gui_display_data_type=Boolean  arith_type=Boolean  n_bits=1  bin_pt=0  float_type=Single  exp_bits=8  fraction_bits=24  quantization=Truncate  overflow=Wrap  en=off  latency=0  dbl_ovrd=off  pipeline=off  block_type=convert
  Convert2 : xbsIndex_r4/Convert  gui_display_data_type=Boolean  arith_type=Boolean  n_bits=1  bin_pt=0  float_type=Single  exp_bits=8  fraction_bits=24  quantization=Truncate  overflow=Wrap  en=off  latency=0  dbl_ovrd=off  pipeline=off  block_type=convert
  Register2 : xbsIndex_r4/Register  init=0  rst=on  en=on  dbl_ovrd=off  block_type=register
      settings: xl_area=[1 1 0 0 0 0 0]  block_version=VER_STRING_GOES_HERE
  Q : Outport
  wires:
  S/1 -> Convert2/1
  Register2/1 -> Q/1
  R/1 -> Convert1/1
  Constant1/1 -> Register2/1
  Convert1/1 -> Register2/2
  Convert2/1 -> Register2/3

== system wlan_phy_rx_pmd/FFT/Sym Count/S-R Latch1 ==
  S : Inport
  R : Inport  Port=2
  Constant1 : xbsIndex_r4/Constant  const=1  gui_display_data_type=Fixed-point  arith_type=Boolean  n_bits=1  bin_pt=0  preci_type=Single  exp_width=8  frac_width=24  explicit_period=off  period=1  equ=P=C  opselect=C  inp2=PCIN>>17  opr=+  inp1=P  carry=CIN  dbl_ovrd=off  block_type=constant
      settings: block_version=VER_STRING_GOES_HERE
  Convert1 : xbsIndex_r4/Convert  gui_display_data_type=Boolean  arith_type=Boolean  n_bits=1  bin_pt=0  float_type=Single  exp_bits=8  fraction_bits=24  quantization=Truncate  overflow=Wrap  en=off  latency=0  dbl_ovrd=off  pipeline=off  block_type=convert
  Convert2 : xbsIndex_r4/Convert  gui_display_data_type=Boolean  arith_type=Boolean  n_bits=1  bin_pt=0  float_type=Single  exp_bits=8  fraction_bits=24  quantization=Truncate  overflow=Wrap  en=off  latency=0  dbl_ovrd=off  pipeline=off  block_type=convert
  Register2 : xbsIndex_r4/Register  init=0  rst=on  en=on  dbl_ovrd=off  block_type=register
      settings: xl_area=[1 1 0 0 0 0 0]  block_version=VER_STRING_GOES_HERE
  Q : Outport
  wires:
  S/1 -> Convert2/1
  Register2/1 -> Q/1
  R/1 -> Convert1/1
  Constant1/1 -> Register2/1
  Convert1/1 -> Register2/2
  Convert2/1 -> Register2/3

== system wlan_phy_rx_pmd/Inputs/RF A Inputs/RF A RSSI Sum ==
  RSSI : Inport
  Down Sample6 : xbsIndex_r4/Down Sample  sample_ratio=16  sample_phase="Last Value of Frame  (most efficient)"  en=off  latency=1  dbl_ovrd=off  block_type=dsamp
      settings: block_version=VER_STRING_GOES_HERE
  RSSI DET Debug Out : Subsystem
  Running Sum : Subsystem
  Sum : Outport
  wires:
  [RX_GLOBAL_RESET] -> Down Sample6/1
  RSSI/1 -> Running Sum/1(RSSI), RSSI DET Debug Out/2(Raw RSSI)
  Down Sample6/1 -> Running Sum/2(reset)
  Running Sum/1(avg) -> Sum/1, RSSI DET Debug Out/1(Avg RSSI)

== system wlan_phy_rx_pmd/Inputs/RF B Inputs/RF B RSSI Sum ==
  RSSI : Inport
  Down Sample6 : xbsIndex_r4/Down Sample  sample_ratio=16  sample_phase="Last Value of Frame  (most efficient)"  en=off  latency=1  dbl_ovrd=off  block_type=dsamp
      settings: block_version=VER_STRING_GOES_HERE
  Running Sum : Subsystem
  Sum : Outport
  wires:
  Running Sum/1(avg) -> Sum/1
  Down Sample6/1 -> Running Sum/2(reset)
  RSSI/1 -> Running Sum/1(RSSI)
  [RX_GLOBAL_RESET] -> Down Sample6/1

== system wlan_phy_rx_pmd/Inputs/RF C Inputs/RF C RSSI Sum ==
  RSSI : Inport
  Down Sample6 : xbsIndex_r4/Down Sample  sample_ratio=16  sample_phase="Last Value of Frame  (most efficient)"  en=off  latency=1  dbl_ovrd=off  block_type=dsamp
      settings: block_version=VER_STRING_GOES_HERE
  Running Sum : Subsystem
  Sum : Outport
  wires:
  Running Sum/1(avg) -> Sum/1
  Down Sample6/1 -> Running Sum/2(reset)
  RSSI/1 -> Running Sum/1(RSSI)
  [RX_GLOBAL_RESET] -> Down Sample6/1

== system wlan_phy_rx_pmd/Inputs/RF D Inputs/RF D RSSI Sum ==
  RSSI : Inport
  Down Sample6 : xbsIndex_r4/Down Sample  sample_ratio=16  sample_phase="Last Value of Frame  (most efficient)"  en=off  latency=1  dbl_ovrd=off  block_type=dsamp
      settings: block_version=VER_STRING_GOES_HERE
  Running Sum : Subsystem
  Sum : Outport
  wires:
  [RX_GLOBAL_RESET] -> Down Sample6/1
  RSSI/1 -> Running Sum/1(RSSI)
  Down Sample6/1 -> Running Sum/2(reset)
  Running Sum/1(avg) -> Sum/1

== system wlan_phy_rx_pmd/MAC Outputs/Pulse Stretch1/S-R Latch ==
  S : Inport
  R : Inport  Port=2
  Constant1 : xbsIndex_r4/Constant  const=1  gui_display_data_type=Boolean  arith_type=Boolean  n_bits=1  bin_pt=0  preci_type=Single  exp_width=8  frac_width=24  explicit_period=off  period=1  equ=P=C  opselect=C  inp2=PCIN>>17  opr=+  inp1=P  carry=CIN  dbl_ovrd=off  block_type=constant
      settings: block_version=VER_STRING_GOES_HERE
  Convert1 : xbsIndex_r4/Convert  gui_display_data_type=Boolean  arith_type=Boolean  n_bits=1  bin_pt=0  float_type=Single  exp_bits=8  fraction_bits=24  quantization=Truncate  overflow=Wrap  en=off  latency=0  dbl_ovrd=off  pipeline=off  block_type=convert
  Convert2 : xbsIndex_r4/Convert  gui_display_data_type=Boolean  arith_type=Boolean  n_bits=1  bin_pt=0  float_type=Single  exp_bits=8  fraction_bits=24  quantization=Truncate  overflow=Wrap  en=off  latency=0  dbl_ovrd=off  pipeline=off  block_type=convert
  Register2 : xbsIndex_r4/Register  init=0  rst=on  en=on  dbl_ovrd=off  block_type=register
      settings: xl_area=[1 1 0 0 0 0 0]  block_version=VER_STRING_GOES_HERE
  Q : Outport
  wires:
  Convert2/1 -> Register2/3
  Convert1/1 -> Register2/2
  Constant1/1 -> Register2/1
  R/1 -> Convert1/1
  Register2/1 -> Q/1
  S/1 -> Convert2/1

== system wlan_phy_rx_pmd/Sync & Antenna Sel/Antenna Selection/Ant Sel Logic ==
  note: [0 0] = RF A
      | [0 1] = RF B
      | [1 0] = RF C
      | [1 1] = RF D
  note: [1 1] = RF A
      | [1 0] = RF B
      | [0 1] = RF C
      | [0 0] = RF D
  G A : Inport
  G B : Inport  Port=2
  G C : Inport  Port=3
  G D : Inport  Port=4
  Concat : xbsIndex_r4/Concat  num_inputs=2  dbl_ovrd=off  block_type=concat
  Inverter2 : xbsIndex_r4/Inverter  en=off  latency=0  dbl_ovrd=off  block_type=inv
  Mux{,1..3} (4 alike) : xbsIndex_r4/Mux  inputs=2  en=off  latency=0  precision=Full  arith_type=Unsigned  n_bits=16  bin_pt=14  quantization=Truncate  overflow=Wrap  dbl_ovrd=off  block_type=mux
  RF A En Mask : Subsystem
  RF B En Mask : Subsystem
  RF C En Mask : Subsystem
  RF D En Mask : Subsystem
  Relational : xbsIndex_r4/Relational  mode=a<=b  en=off  latency=0  dbl_ovrd=off  block_type=relational
  Relational1 : xbsIndex_r4/Relational  mode=a<=b  en=off  latency=0  dbl_ovrd=off  block_type=relational
  Relational2 : xbsIndex_r4/Relational  mode=a<=b  en=off  latency=0  dbl_ovrd=off  block_type=relational
  Ant Sel : Outport
  wires:
  RF D En Mask/1(" GD") -> Relational1/2, Mux1/2
  RF C En Mask/1(" GC") -> Relational1/1, Mux1/3
  RF B En Mask/1(" GB") -> Relational/2, Mux/2
  RF A En Mask/1(" GA") -> Relational/1, Mux/3
  Relational/1 -> Mux/1, Mux2/3
  Mux/1 -> Relational2/1
  Relational1/1 -> Mux1/1, Mux2/2
  Mux1/1 -> Relational2/2
  Relational2/1 -> Concat/1, Mux2/1
  Mux2/1 -> Concat/2
  Concat/1 -> Inverter2/1
  Inverter2/1 -> Mux3/3
  [regRx_switchingDiv_En] -> Mux3/1
  G A/1 -> RF A En Mask/1(GA)
  G B/1 -> RF B En Mask/1(GB)
  G C/1 -> RF C En Mask/1(GC)
  G D/1 -> RF D En Mask/1(GD)
  [regRx_noSwitching_AntSel] -> Mux3/2
  Mux3/1 -> Ant Sel/1

== system wlan_phy_rx_pmd/Sync & Antenna Sel/Antenna Selection/Posedge ==
  note: Rising Edge Detection
  D : Inport
  Delay : xbsIndex_r4/Delay  rst=off  infoeditControl=Selection of Reset will increase slice count due to use of real FFs and instead of SRLs  en=off  latency=1  dbl_ovrd=off  reg_retiming=off  block_type=delay
      settings: infoedit=Hardware notes: A delay line is a chain, each link of which is an SRL16 followed by a flip-flop. If register retiming is enabled, the delay line is a chain of flip-flops.  xl_area=[1 1 0 0 0 0 0]  block_version=VER_STRING_GOES_HERE
  Inverter : xbsIndex_r4/Inverter  en=off  latency=0  dbl_ovrd=off  block_type=inv
      settings: xl_area=[0 0 0 0 0 0 0]  block_version=VER_STRING_GOES_HERE
  Logical1 : xbsIndex_r4/Logical  logical_function=AND  inputs=2  en=off  latency=0  precision=Full  arith_type=Unsigned  n_bits=8  bin_pt=2  align_bp=on  dbl_ovrd=off  block_type=logical
      settings: xl_area=[1 0 0 1 0 0 0]  block_version=VER_STRING_GOES_HERE
  Q : Outport
  wires:
  D/1 -> Logical1/2, Delay/1
  Logical1/1 -> Q/1
  Inverter/1 -> Logical1/1
  Delay/1 -> Inverter/1

== system wlan_phy_rx_pmd/Sync & Antenna Sel/Antenna Selection/RF A Gains ==
  Capt : Inport
  Rst : Inport  Port=2
  Concat : xbsIndex_r4/Concat  num_inputs=2  dbl_ovrd=off  block_type=concat
  Register2 : xbsIndex_r4/Register  init=0  rst=on  en=on  dbl_ovrd=off  block_type=register
      settings: xl_area=[1 1 0 0 0 0 0]  block_version=VER_STRING_GOES_HERE
  G_vec : Outport
  wires:
  [RFA_AGC_G_RF] -> Concat/1
  [RFA_AGC_G_BB] -> Concat/2
  Concat/1 -> Register2/1
  Rst/1 -> Register2/2
  Capt/1 -> Register2/3
  Register2/1 -> G_vec/1

== system wlan_phy_rx_pmd/Sync & Antenna Sel/Antenna Selection/RF B Gains ==
  Capt : Inport
  Rst : Inport  Port=2
  Concat : xbsIndex_r4/Concat  num_inputs=2  dbl_ovrd=off  block_type=concat
  Register2 : xbsIndex_r4/Register  init=0  rst=on  en=on  dbl_ovrd=off  block_type=register
      settings: xl_area=[1 1 0 0 0 0 0]  block_version=VER_STRING_GOES_HERE
  G_vec : Outport
  wires:
  Register2/1 -> G_vec/1
  Capt/1 -> Register2/3
  Rst/1 -> Register2/2
  Concat/1 -> Register2/1
  [RFB_AGC_G_BB] -> Concat/2
  [RFB_AGC_G_RF] -> Concat/1

== system wlan_phy_rx_pmd/Sync & Antenna Sel/Antenna Selection/RF C Gains ==
  Capt : Inport
  Rst : Inport  Port=2
  Concat : xbsIndex_r4/Concat  num_inputs=2  dbl_ovrd=off  block_type=concat
  Register2 : xbsIndex_r4/Register  init=0  rst=on  en=on  dbl_ovrd=off  block_type=register
      settings: xl_area=[1 1 0 0 0 0 0]  block_version=VER_STRING_GOES_HERE
  G_vec : Outport
  wires:
  Register2/1 -> G_vec/1
  Capt/1 -> Register2/3
  Rst/1 -> Register2/2
  Concat/1 -> Register2/1
  [RFC_AGC_G_BB] -> Concat/2
  [RFC_AGC_G_RF] -> Concat/1

== system wlan_phy_rx_pmd/Sync & Antenna Sel/Antenna Selection/RF D Gains ==
  Capt : Inport
  Rst : Inport  Port=2
  Concat : xbsIndex_r4/Concat  num_inputs=2  dbl_ovrd=off  block_type=concat
  Register2 : xbsIndex_r4/Register  init=0  rst=on  en=on  dbl_ovrd=off  block_type=register
      settings: xl_area=[1 1 0 0 0 0 0]  block_version=VER_STRING_GOES_HERE
  G_vec : Outport
  wires:
  [RFD_AGC_G_RF] -> Concat/1
  [RFD_AGC_G_BB] -> Concat/2
  Concat/1 -> Register2/1
  Rst/1 -> Register2/2
  Capt/1 -> Register2/3
  Register2/1 -> G_vec/1

== system wlan_phy_rx_pmd/Sync & Antenna Sel/Antenna Selection/RSSI & Gain Capture ==
  note: Capture RSSI after AGC has settled
      | (avoids saturating RSSI when G_RF=3 and Rx power is high)
      | Software must compensate for RF gain selection when
      | interpretting thse RSSI values.
  note: Capture gains for all antennas
      | after AGC has settled.
  RSSI Vec : Inport
  16MSB{,1..3} (4 alike) : xbsIndex_r4/Slice  nbits=16  boolean_output=off  mode=Upper Bit Location + Width  bit1=0  base1=MSB of Input  bit0=0  base0=LSB of Input  dbl_ovrd=off  block_type=slice
  Bus Selector3 : BusSelector  OutputSignals=RFB_RSSI,RFA_RSSI,RFD_RSSI,RFC_RSSI
  Concat{,2..4,6..7} (6 alike) : xbsIndex_r4/Concat  num_inputs=2  dbl_ovrd=off  block_type=concat
  Concat1 : xbsIndex_r4/Concat  num_inputs=7  dbl_ovrd=off  block_type=concat
  Constant1 : xbsIndex_r4/Constant  const=0  gui_display_data_type=Fixed-point  arith_type=Unsigned  n_bits=1  bin_pt=0  preci_type=Single  exp_width=8  frac_width=24  explicit_period=off  period=1  equ=P=C  opselect=C  inp2=PCIN>>17  opr=+  inp1=P  carry=CIN  dbl_ovrd=off  block_type=constant
      settings: block_version=VER_STRING_GOES_HERE
  Delay1 : xbsIndex_r4/Delay  rst=off  infoeditControl=Selection of Reset will increase slice count due to use of real FFs and instead of SRLs  en=off  latency=4  dbl_ovrd=off  reg_retiming=off  block_type=delay
  Posedge1 : Subsystem
  Register1 : xbsIndex_r4/Register  init=0  rst=off  en=on  dbl_ovrd=off  block_type=register
  Register2 : xbsIndex_r4/Register  init=0  rst=off  en=on  dbl_ovrd=off  block_type=register
  Register{3,5..9} (6 alike) : xbsIndex_r4/Register  init=0  rst=off  en=off  dbl_ovrd=off  block_type=register
  Register4 : xbsIndex_r4/Register  init=0  rst=off  en=on  dbl_ovrd=off  block_type=register
  Up Sample : xbsIndex_r4/Up Sample  sample_ratio=16  copy_samples=on  en=off  latency=0  dbl_ovrd=off  block_type=usamp
  Up Sample1 : xbsIndex_r4/Up Sample  sample_ratio=16  copy_samples=on  en=off  latency=0  dbl_ovrd=off  block_type=usamp
  tag scope: GAINS_POST_AGC=global, RSSI_POST_AGC_AB=global, RSSI_POST_AGC_CD=global
  wires:
  [RFA_AGC_G_BB] -> Concat/2
  Register4/1 -> Register5/1
  Up Sample1/1 -> Register4/1
  Concat4/1 -> Up Sample1/1
  Concat6/1 -> Concat1/3
  Concat7/1 -> Concat1/1
  [RFD_AGC_G_RF] -> Concat7/1
  [RFD_AGC_G_BB] -> Concat7/2
  [RFC_AGC_G_BB] -> Concat6/2
  [RFC_AGC_G_RF] -> Concat6/1
  Concat3/1 -> Concat1/5
  Concat/1 -> Concat1/7
  Constant1/1 -> Concat1/6, Concat1/4, Concat1/2
  [RFB_AGC_G_RF] -> Concat3/1
  [RFB_AGC_G_BB] -> Concat3/2
  [RFA_AGC_G_RF] -> Concat/1
  Posedge1/1(Q) -> Delay1/1
  [AGC_DONE] -> Posedge1/1(D)
  16MSB1/1 -> Concat2/2
  16MSB/1 -> Concat2/1
  Up Sample/1 -> Register1/1
  Concat2/1 -> Up Sample/1
  Register1/1 -> Register3/1
  Register2/1 -> Register6/1
  Concat1/1 -> Register2/1
  RSSI Vec/1 -> Bus Selector3/1
  16MSB2/1 -> Concat4/1
  16MSB3/1 -> Concat4/2
  Delay1/1 -> Register1/2, Register4/2, Register2/2
  Bus Selector3/1 -> 16MSB/1  "<RFB_RSSI>"
  Bus Selector3/2 -> 16MSB1/1  "<RFA_RSSI>"
  Bus Selector3/3 -> 16MSB2/1  "<RFD_RSSI>"
  Bus Selector3/4 -> 16MSB3/1  "<RFC_RSSI>"
  Register3/1 -> Register9/1
  Register5/1 -> Register8/1
  Register6/1 -> Register7/1
  Register7/1 -> [GAINS_POST_AGC]
  Register8/1 -> [RSSI_POST_AGC_CD]
  Register9/1 -> [RSSI_POST_AGC_AB]

== system wlan_phy_rx_pmd/Sync & Antenna Sel/Debug  Outputs/Pulse Stretch ==
  P : Inport
  Delay : xbsIndex_r4/Delay  rst=off  infoeditControl=Selection of Reset will increase slice count due to use of real FFs and instead of SRLs  en=off  latency=16  dbl_ovrd=off  reg_retiming=off  block_type=delay
  S-R Latch : Subsystem
  P16 : Outport
  wires:
  S-R Latch/1(Q) -> Delay/1, P16/1
  Delay/1 -> S-R Latch/2(R)
  P/1 -> S-R Latch/1(S)

== system wlan_phy_rx_pmd/Sync & Antenna Sel/Debug  Outputs/Pulse Stretch1 ==
  P : Inport
  Delay : xbsIndex_r4/Delay  rst=off  infoeditControl=Selection of Reset will increase slice count due to use of real FFs and instead of SRLs  en=off  latency=16  dbl_ovrd=off  reg_retiming=off  block_type=delay
  S-R Latch : Subsystem
  P16 : Outport
  wires:
  S-R Latch/1(Q) -> Delay/1, P16/1
  Delay/1 -> S-R Latch/2(R)
  P/1 -> S-R Latch/1(S)
